simulink library "lib_all"  (Simulink 7.6)
settings: Solver=ode45  SolverMode=SingleTasking  StartTime=0.0  StopTime=10.0  RelTol=1e-3
values from neighbouring files (symbols this model uses):
  map_lib_03 = [6.8679 7.0159 7.4571 8.1849 9.1892 10.456 11.97 13.71 15.655 17.781 20.063 22.474 ... (803 elements, 73x11)]    [scr_simdata.mat]    (also in scr_simdata_all.mat)
  map_lib_06 = [2.5784 2.6727 2.9588 3.4453 4.1444 5.068 6.226 7.6242 9.2634 11.139 13.24 15.552 ... (803 elements, 73x11)]    [scr_simdata.mat]    (also in scr_simdata_all.mat)
  map_lib_09 = [0.0025784 0.0026727 0.0029588 0.0034453 0.0041444 0.005068 0.006226 0.0076242 0.0092634 0.011139 0.01324 0.015552 ... (803 elements, 73x11)]    [scr_simdata.mat]    (also in scr_simdata_all.mat)
  map_lib_10 = [2.74 2.75 2.76 2.77 2.78 2.79 2.8 2.81 2.82 2.83 2.84 2.85 ... (81 elements, 1x81)]    [scr_simdata.mat]    (also in scr_simdata_all.mat)
  map_lib_11 = [1.1983 1.2027 1.207 1.2113 1.2156 1.2198 1.2241 1.2283 1.2326 1.2368 1.241 1.2451 ... (81 elements, 1x81)]    [scr_simdata.mat]    (also in scr_simdata_all.mat)
  map_lib_12 = [2.74 2.75 2.76 2.77 2.78 2.79 2.8 2.81 2.82 2.83 2.84 2.85 ... (81 elements, 1x81)]    [scr_simdata.mat]    (also in scr_simdata_all.mat)
  map_lib_13 = [1.74 1.75 1.76 1.77 1.78 1.79 1.8 1.81 1.82 1.83 1.84 1.85 ... (81 elements, 1x81)]    [scr_simdata.mat]    (also in scr_simdata_all.mat)
  map_lib_16 = [0.00048828125 1.6159 1.8962 2.0821 2.2251 2.3426 2.4433 2.5318 2.611 2.683 2.749 2.8101 ... (1419 elements, 129x11)]    [scr_simdata.mat]    (also in scr_simdata_all.mat)
  map_mr2_01 = [0 800 1600 2400 3200 4000]    [scr_simdata.mat]    (also in scr_simdata_all.mat)
  map_mr2_02 = [1.32 1.32 1.33 1.34 1.36 1.38]    [scr_simdata.mat]    (also in scr_simdata_all.mat)
  map_mr2_03 = [0 800 1600 2400 3200 4000]    [scr_simdata.mat]    (also in scr_simdata_all.mat)
  map_mr2_04 = [1.32 1.32 1.33 1.34 1.36 1.38]    [scr_simdata.mat]    (also in scr_simdata_all.mat)
  map_mr2_05 = [0 800 1600 2400 3200 4000]    [scr_simdata.mat]    (also in scr_simdata_all.mat)
  map_mr2_06 = [1.32 1.32 1.33 1.34 1.36 1.38]    [scr_simdata.mat]    (also in scr_simdata_all.mat)
  Parameter1 = 6    [scr_simdata.mat]    (also in scr_simdata_all.mat)
  Parameter101 = 30    [scr_simdata.mat]    (also in scr_simdata_all.mat)
  Parameter102 = 12    [scr_simdata.mat]    (also in scr_simdata_all.mat)
  Parameter103 = -240    [scr_simdata.mat]    (also in scr_simdata_all.mat)
  Parameter108 = 48    [scr_simdata.mat]    (also in scr_simdata_all.mat)
  Parameter120 = 16    [scr_simdata.mat]    (also in scr_simdata_all.mat)
  Parameter121 = 87    [scr_simdata.mat]    (also in scr_simdata_all.mat)
  Parameter123 = 78    [scr_simdata.mat]    (also in scr_simdata_all.mat)
  Parameter124 = 60    [scr_simdata.mat]    (also in scr_simdata_all.mat)
  Parameter125 = -480    [scr_simdata.mat]    (also in scr_simdata_all.mat)
  Parameter130 = 96    [scr_simdata.mat]    (also in scr_simdata_all.mat)
  Parameter131 = 3    [scr_simdata.mat]    (also in scr_simdata_all.mat)
  Parameter144 = 3    [scr_simdata.mat]    (also in scr_simdata_all.mat)
  Parameter18 = 128    [scr_simdata.mat]    (also in scr_simdata_all.mat)
  Parameter19 = 16    [scr_simdata.mat]    (also in scr_simdata_all.mat)
  Parameter2 = 12    [scr_simdata.mat]    (also in scr_simdata_all.mat)
  Parameter20 = 7    [scr_simdata.mat]    (also in scr_simdata_all.mat)
  Parameter21 = 4    [scr_simdata.mat]    (also in scr_simdata_all.mat)
  Parameter3 = 18    [scr_simdata.mat]    (also in scr_simdata_all.mat)
  Parameter32 = 1    [scr_simdata.mat]    (also in scr_simdata_all.mat)
  Parameter34 = 24    [scr_simdata.mat]    (also in scr_simdata_all.mat)
  Parameter4 = 138    [scr_simdata.mat]    (also in scr_simdata_all.mat)
  Parameter42 = 48    [scr_simdata.mat]    (also in scr_simdata_all.mat)
  Parameter5 = 135    [scr_simdata.mat]    (also in scr_simdata_all.mat)
  Parameter55 = 0    [scr_simdata.mat]    (also in scr_simdata_all.mat)
  Parameter56 = 0    [scr_simdata.mat]    (also in scr_simdata_all.mat)
  Parameter57 = 1    [scr_simdata.mat]    (also in scr_simdata_all.mat)
  Parameter58 = 1    [scr_simdata.mat]    (also in scr_simdata_all.mat)
  Parameter59 = 2    [scr_simdata.mat]    (also in scr_simdata_all.mat)
  Parameter6 = 132    [scr_simdata.mat]    (also in scr_simdata_all.mat)
  Parameter60 = 2    [scr_simdata.mat]    (also in scr_simdata_all.mat)
  Parameter63 = 144    [scr_simdata.mat]    (also in scr_simdata_all.mat)
  Parameter7 = 126    [scr_simdata.mat]    (also in scr_simdata_all.mat)
  Parameter76 = 64    [scr_simdata.mat]    (also in scr_simdata_all.mat)
  Parameter77 = 135    [scr_simdata.mat]    (also in scr_simdata_all.mat)
  Parameter79 = 126    [scr_simdata.mat]    (also in scr_simdata_all.mat)
  Parameter80 = 108    [scr_simdata.mat]    (also in scr_simdata_all.mat)
  Parameter81 = 0    [scr_simdata.mat]    (also in scr_simdata_all.mat)
  Parameter86 = 0    [scr_simdata.mat]    (also in scr_simdata_all.mat)
  Parameter98 = 112    [scr_simdata.mat]    (also in scr_simdata_all.mat)
  Parameter99 = 39    [scr_simdata.mat]    (also in scr_simdata_all.mat)
undefined symbols (not defined in the file or in its folder): cylvolkp_hih_map2h, cylvolkp_map2h, pk1k_map2h
libraries: simulink x2
(part 18 of 36)

== system lib_all/SubSystem1364/SubSystem1419/SubSystem1428/SubSystem1452/SubSystem1453 ==
  note: ***編集禁止***
  element : Inport
  Inside_DSR : DataStoreRead  DataStoreName=DSM5
  Selector : Selector  IndexOptions=Index vector (port)  Indices=[1 3]  OutputSizes=1
  Output : Outport
  wires:
  Inside_DSR/1 -> Selector/1
  element/1 -> Selector/2
  Selector/1 -> Output/1

== system lib_all/SubSystem1364/SubSystem1419/SubSystem1428/SubSystem1452/SubSystem1454 ==
  Block3948 : Inport
  Block3949 : Inport  Port=2
  Block3950 : MultiPortSwitch  zeroidx=off  RndMeth=Zero  SaturateOnIntegerOverflow=on  InputSameDT=on
  SubSystem1455 : Subsystem  TreatAsAtomicUnit=on  mask(***ATC.DSRVEC***){bd_version=1.01.A, r0=DSM7, r1=-1, r2=, r3=, r4=, r5=, r6=, r7=, r8=, r9=, c0=, c1=, c2=, c3=, c4=, c5=, c6=, c7=, c8=, c9=}
      mask prompts: bd_version "Version"
      mask help: これはAutoCからのブロックです．編集禁止．
      settings: OpenFcn=warndlg('変更禁止!');
      mask Help: web(ui_blockhelpmng(gcb));
      mask Display: disp('ATC.DSRVEC')
  SubSystem1456 : Subsystem  TreatAsAtomicUnit=on  mask(***ATC.DSRVEC***){bd_version=1.01.A, r0=DSM6, r1=-1, r2=, r3=, r4=, r5=, r6=, r7=, r8=, r9=, c0=, c1=, c2=, c3=, c4=, c5=, c6=, c7=, c8=, c9=}
      mask prompts: bd_version "Version"
      mask help: これはAutoCからのブロックです．編集禁止．
      settings: OpenFcn=warndlg('変更禁止!');
      mask Help: web(ui_blockhelpmng(gcb));
      mask Display: disp('ATC.DSRVEC')
  SubSystem1457 : Subsystem  TreatAsAtomicUnit=on  mask(***ATC.DSRVEC***){bd_version=1.01.A, r0=DSM19, r1=-1, r2=, r3=, r4=, r5=, r6=, r7=, r8=, r9=, c0=, c1=, c2=, c3=, c4=, c5=, c6=, c7=, c8=, c9=}
      mask prompts: bd_version "Version"
      mask help: これはAutoCからのブロックです．編集禁止．
      settings: OpenFcn=warndlg('変更禁止!');
      mask Help: web(ui_blockhelpmng(gcb));
      mask Display: disp('ATC.DSRVEC')
  Block3951 : Outport
  wires:
  Block3949/1 -> SubSystem1457/1(element), SubSystem1456/1(element), SubSystem1455/1(element)
  SubSystem1457/1(Output) -> Block3950/4
  SubSystem1456/1(Output) -> Block3950/3
  SubSystem1455/1(Output) -> Block3950/2
  Block3950/1 -> Block3951/1
  Block3948/1 -> Block3950/1

== system lib_all/SubSystem1364/SubSystem1419/SubSystem1428/SubSystem1452/SubSystem1458 ==
  Block3952 : Inport
  Block3953 : Inport  Port=2
  Block3954 : MultiPortSwitch  zeroidx=off  RndMeth=Zero  SaturateOnIntegerOverflow=on  InputSameDT=on
  SubSystem1459 : Subsystem  TreatAsAtomicUnit=on  mask(***ATC.DSRVEC***){bd_version=1.01.A, r0=DSM7, r1=-1, r2=, r3=, r4=, r5=, r6=, r7=, r8=, r9=, c0=, c1=, c2=, c3=, c4=, c5=, c6=, c7=, c8=, c9=}
      mask prompts: bd_version "Version"
      mask help: これはAutoCからのブロックです．編集禁止．
      settings: OpenFcn=warndlg('変更禁止!');
      mask Help: web(ui_blockhelpmng(gcb));
      mask Display: disp('ATC.DSRVEC')
  SubSystem1460 : Subsystem  TreatAsAtomicUnit=on  mask(***ATC.DSRVEC***){bd_version=1.01.A, r0=DSM6, r1=-1, r2=, r3=, r4=, r5=, r6=, r7=, r8=, r9=, c0=, c1=, c2=, c3=, c4=, c5=, c6=, c7=, c8=, c9=}
      mask prompts: bd_version "Version"
      mask help: これはAutoCからのブロックです．編集禁止．
      settings: OpenFcn=warndlg('変更禁止!');
      mask Help: web(ui_blockhelpmng(gcb));
      mask Display: disp('ATC.DSRVEC')
  SubSystem1461 : Subsystem  TreatAsAtomicUnit=on  mask(***ATC.DSRVEC***){bd_version=1.01.A, r0=DSM19, r1=-1, r2=, r3=, r4=, r5=, r6=, r7=, r8=, r9=, c0=, c1=, c2=, c3=, c4=, c5=, c6=, c7=, c8=, c9=}
      mask prompts: bd_version "Version"
      mask help: これはAutoCからのブロックです．編集禁止．
      settings: OpenFcn=warndlg('変更禁止!');
      mask Help: web(ui_blockhelpmng(gcb));
      mask Display: disp('ATC.DSRVEC')
  Block3955 : Outport
  wires:
  Block3953/1 -> SubSystem1461/1(element), SubSystem1460/1(element), SubSystem1459/1(element)
  SubSystem1461/1(Output) -> Block3954/4
  SubSystem1460/1(Output) -> Block3954/3
  SubSystem1459/1(Output) -> Block3954/2
  Block3954/1 -> Block3955/1
  Block3952/1 -> Block3954/1

== system lib_all/SubSystem1364/SubSystem1419/SubSystem1428/SubSystem1462/SubSystem1463 ==
  note: ***編集禁止***
  element : Inport
  Inside_DSR : DataStoreRead  DataStoreName=DSM3
  Selector : Selector  IndexOptions=Index vector (port)  Indices=[1 3]  OutputSizes=1
  Output : Outport
  wires:
  Inside_DSR/1 -> Selector/1
  element/1 -> Selector/2
  Selector/1 -> Output/1

== system lib_all/SubSystem1364/SubSystem1419/SubSystem1428/SubSystem1462/SubSystem1464 ==
  Block3963 : Inport
  Block3964 : Inport  Port=2
  Block3965 : MultiPortSwitch  zeroidx=off  RndMeth=Zero  SaturateOnIntegerOverflow=on  InputSameDT=on
  SubSystem1465 : Subsystem  TreatAsAtomicUnit=on  mask(***ATC.DSRVEC***){bd_version=1.01.A, r0=DSM7, r1=-1, r2=, r3=, r4=, r5=, r6=, r7=, r8=, r9=, c0=, c1=, c2=, c3=, c4=, c5=, c6=, c7=, c8=, c9=}
      mask prompts: bd_version "Version"
      mask help: これはAutoCからのブロックです．編集禁止．
      settings: OpenFcn=warndlg('変更禁止!');
      mask Help: web(ui_blockhelpmng(gcb));
      mask Display: disp('ATC.DSRVEC')
  SubSystem1466 : Subsystem  TreatAsAtomicUnit=on  mask(***ATC.DSRVEC***){bd_version=1.01.A, r0=DSM6, r1=-1, r2=, r3=, r4=, r5=, r6=, r7=, r8=, r9=, c0=, c1=, c2=, c3=, c4=, c5=, c6=, c7=, c8=, c9=}
      mask prompts: bd_version "Version"
      mask help: これはAutoCからのブロックです．編集禁止．
      settings: OpenFcn=warndlg('変更禁止!');
      mask Help: web(ui_blockhelpmng(gcb));
      mask Display: disp('ATC.DSRVEC')
  SubSystem1467 : Subsystem  TreatAsAtomicUnit=on  mask(***ATC.DSRVEC***){bd_version=1.01.A, r0=DSM19, r1=-1, r2=, r3=, r4=, r5=, r6=, r7=, r8=, r9=, c0=, c1=, c2=, c3=, c4=, c5=, c6=, c7=, c8=, c9=}
      mask prompts: bd_version "Version"
      mask help: これはAutoCからのブロックです．編集禁止．
      settings: OpenFcn=warndlg('変更禁止!');
      mask Help: web(ui_blockhelpmng(gcb));
      mask Display: disp('ATC.DSRVEC')
  Block3966 : Outport
  wires:
  Block3964/1 -> SubSystem1467/1(element), SubSystem1466/1(element), SubSystem1465/1(element)
  SubSystem1467/1(Output) -> Block3965/4
  SubSystem1466/1(Output) -> Block3965/3
  SubSystem1465/1(Output) -> Block3965/2
  Block3965/1 -> Block3966/1
  Block3963/1 -> Block3965/1

== system lib_all/SubSystem1364/SubSystem1419/SubSystem1428/SubSystem1462/SubSystem1468 ==
  Block3967 : Inport
  Block3968 : Inport  Port=2
  Block3969 : MultiPortSwitch  zeroidx=off  RndMeth=Zero  SaturateOnIntegerOverflow=on  InputSameDT=on
  SubSystem1469 : Subsystem  TreatAsAtomicUnit=on  mask(***ATC.DSRVEC***){bd_version=1.01.A, r0=DSM7, r1=-1, r2=, r3=, r4=, r5=, r6=, r7=, r8=, r9=, c0=, c1=, c2=, c3=, c4=, c5=, c6=, c7=, c8=, c9=}
      mask prompts: bd_version "Version"
      mask help: これはAutoCからのブロックです．編集禁止．
      settings: OpenFcn=warndlg('変更禁止!');
      mask Help: web(ui_blockhelpmng(gcb));
      mask Display: disp('ATC.DSRVEC')
  SubSystem1470 : Subsystem  TreatAsAtomicUnit=on  mask(***ATC.DSRVEC***){bd_version=1.01.A, r0=DSM6, r1=-1, r2=, r3=, r4=, r5=, r6=, r7=, r8=, r9=, c0=, c1=, c2=, c3=, c4=, c5=, c6=, c7=, c8=, c9=}
      mask prompts: bd_version "Version"
      mask help: これはAutoCからのブロックです．編集禁止．
      settings: OpenFcn=warndlg('変更禁止!');
      mask Help: web(ui_blockhelpmng(gcb));
      mask Display: disp('ATC.DSRVEC')
  SubSystem1471 : Subsystem  TreatAsAtomicUnit=on  mask(***ATC.DSRVEC***){bd_version=1.01.A, r0=DSM19, r1=-1, r2=, r3=, r4=, r5=, r6=, r7=, r8=, r9=, c0=, c1=, c2=, c3=, c4=, c5=, c6=, c7=, c8=, c9=}
      mask prompts: bd_version "Version"
      mask help: これはAutoCからのブロックです．編集禁止．
      settings: OpenFcn=warndlg('変更禁止!');
      mask Help: web(ui_blockhelpmng(gcb));
      mask Display: disp('ATC.DSRVEC')
  Block3970 : Outport
  wires:
  Block3968/1 -> SubSystem1471/1(element), SubSystem1470/1(element), SubSystem1469/1(element)
  SubSystem1471/1(Output) -> Block3969/4
  SubSystem1470/1(Output) -> Block3969/3
  SubSystem1469/1(Output) -> Block3969/2
  Block3969/1 -> Block3970/1
  Block3967/1 -> Block3969/1

== system lib_all/SubSystem1487/SubSystem1496/SubSystem1501/SubSystem1502/SubSystem1503 ==
  note: ***編集禁止***
  element : Inport
  Inside_DSR : DataStoreRead  DataStoreName=DSM7
  Selector : Selector  IndexOptions=Index vector (port)  Indices=[1 3]  OutputSizes=1
  Output : Outport
  wires:
  Selector/1 -> Output/1
  element/1 -> Selector/2
  Inside_DSR/1 -> Selector/1

== system lib_all/SubSystem1487/SubSystem1496/SubSystem1501/SubSystem1502/SubSystem1504 ==
  note: ***編集禁止***
  element : Inport
  Inside_DSR : DataStoreRead  DataStoreName=DSM6
  Selector : Selector  IndexOptions=Index vector (port)  Indices=[1 3]  OutputSizes=1
  Output : Outport
  wires:
  Inside_DSR/1 -> Selector/1
  element/1 -> Selector/2
  Selector/1 -> Output/1

== system lib_all/SubSystem1487/SubSystem1496/SubSystem1501/SubSystem1502/SubSystem1505 ==
  note: ***編集禁止***
  element : Inport
  Inside_DSR : DataStoreRead  DataStoreName=DSM19
  Selector : Selector  IndexOptions=Index vector (port)  Indices=[1 3]  OutputSizes=1
  Output : Outport
  wires:
  Selector/1 -> Output/1
  element/1 -> Selector/2
  Inside_DSR/1 -> Selector/1

== system lib_all/SubSystem1487/SubSystem1496/SubSystem1501/SubSystem1506/SubSystem1507 ==
  note: ***編集禁止***
  element : Inport
  Inside_DSR : DataStoreRead  DataStoreName=DSM7
  Selector : Selector  IndexOptions=Index vector (port)  Indices=[1 3]  OutputSizes=1
  Output : Outport
  wires:
  Selector/1 -> Output/1
  element/1 -> Selector/2
  Inside_DSR/1 -> Selector/1

== system lib_all/SubSystem1487/SubSystem1496/SubSystem1501/SubSystem1506/SubSystem1508 ==
  note: ***編集禁止***
  element : Inport
  Inside_DSR : DataStoreRead  DataStoreName=DSM6
  Selector : Selector  IndexOptions=Index vector (port)  Indices=[1 3]  OutputSizes=1
  Output : Outport
  wires:
  Inside_DSR/1 -> Selector/1
  element/1 -> Selector/2
  Selector/1 -> Output/1

== system lib_all/SubSystem1487/SubSystem1496/SubSystem1501/SubSystem1506/SubSystem1509 ==
  note: ***編集禁止***
  element : Inport
  Inside_DSR : DataStoreRead  DataStoreName=DSM19
  Selector : Selector  IndexOptions=Index vector (port)  Indices=[1 3]  OutputSizes=1
  Output : Outport
  wires:
  Selector/1 -> Output/1
  element/1 -> Selector/2
  Inside_DSR/1 -> Selector/1

== system lib_all/SubSystem1487/SubSystem1496/SubSystem1510/SubSystem1512/SubSystem1513 ==
  note: ***編集禁止***
  element : Inport
  Inside_DSR : DataStoreRead  DataStoreName=DSM7
  Selector : Selector  IndexOptions=Index vector (port)  Indices=[1 3]  OutputSizes=1
  Output : Outport
  wires:
  Selector/1 -> Output/1
  element/1 -> Selector/2
  Inside_DSR/1 -> Selector/1

== system lib_all/SubSystem1487/SubSystem1496/SubSystem1510/SubSystem1512/SubSystem1514 ==
  note: ***編集禁止***
  element : Inport
  Inside_DSR : DataStoreRead  DataStoreName=DSM6
  Selector : Selector  IndexOptions=Index vector (port)  Indices=[1 3]  OutputSizes=1
  Output : Outport
  wires:
  Inside_DSR/1 -> Selector/1
  element/1 -> Selector/2
  Selector/1 -> Output/1

== system lib_all/SubSystem1487/SubSystem1496/SubSystem1510/SubSystem1512/SubSystem1515 ==
  note: ***編集禁止***
  element : Inport
  Inside_DSR : DataStoreRead  DataStoreName=DSM19
  Selector : Selector  IndexOptions=Index vector (port)  Indices=[1 3]  OutputSizes=1
  Output : Outport
  wires:
  Selector/1 -> Output/1
  element/1 -> Selector/2
  Inside_DSR/1 -> Selector/1

== system lib_all/SubSystem1487/SubSystem1496/SubSystem1510/SubSystem1516/SubSystem1517 ==
  note: ***編集禁止***
  element : Inport
  Inside_DSR : DataStoreRead  DataStoreName=DSM7
  Selector : Selector  IndexOptions=Index vector (port)  Indices=[1 3]  OutputSizes=1
  Output : Outport
  wires:
  Selector/1 -> Output/1
  element/1 -> Selector/2
  Inside_DSR/1 -> Selector/1

== system lib_all/SubSystem1487/SubSystem1496/SubSystem1510/SubSystem1516/SubSystem1518 ==
  note: ***編集禁止***
  element : Inport
  Inside_DSR : DataStoreRead  DataStoreName=DSM6
  Selector : Selector  IndexOptions=Index vector (port)  Indices=[1 3]  OutputSizes=1
  Output : Outport
  wires:
  Inside_DSR/1 -> Selector/1
  element/1 -> Selector/2
  Selector/1 -> Output/1

== system lib_all/SubSystem1487/SubSystem1496/SubSystem1510/SubSystem1516/SubSystem1519 ==
  note: ***編集禁止***
  element : Inport
  Inside_DSR : DataStoreRead  DataStoreName=DSM19
  Selector : Selector  IndexOptions=Index vector (port)  Indices=[1 3]  OutputSizes=1
  Output : Outport
  wires:
  Selector/1 -> Output/1
  element/1 -> Selector/2
  Inside_DSR/1 -> Selector/1

== system lib_all/SubSystem1487/SubSystem1496/SubSystem1520/SubSystem1522/SubSystem1523 ==
  note: ***編集禁止***
  element : Inport
  Inside_DSR : DataStoreRead  DataStoreName=DSM7
  Selector : Selector  IndexOptions=Index vector (port)  Indices=[1 3]  OutputSizes=1
  Output : Outport
  wires:
  Selector/1 -> Output/1
  element/1 -> Selector/2
  Inside_DSR/1 -> Selector/1

== system lib_all/SubSystem1487/SubSystem1496/SubSystem1520/SubSystem1522/SubSystem1524 ==
  note: ***編集禁止***
  element : Inport
  Inside_DSR : DataStoreRead  DataStoreName=DSM6
  Selector : Selector  IndexOptions=Index vector (port)  Indices=[1 3]  OutputSizes=1
  Output : Outport
  wires:
  Inside_DSR/1 -> Selector/1
  element/1 -> Selector/2
  Selector/1 -> Output/1

== system lib_all/SubSystem1487/SubSystem1496/SubSystem1520/SubSystem1522/SubSystem1525 ==
  note: ***編集禁止***
  element : Inport
  Inside_DSR : DataStoreRead  DataStoreName=DSM19
  Selector : Selector  IndexOptions=Index vector (port)  Indices=[1 3]  OutputSizes=1
  Output : Outport
  wires:
  Selector/1 -> Output/1
  element/1 -> Selector/2
  Inside_DSR/1 -> Selector/1

== system lib_all/SubSystem1487/SubSystem1496/SubSystem1520/SubSystem1526/SubSystem1527 ==
  note: ***編集禁止***
  element : Inport
  Inside_DSR : DataStoreRead  DataStoreName=DSM7
  Selector : Selector  IndexOptions=Index vector (port)  Indices=[1 3]  OutputSizes=1
  Output : Outport
  wires:
  Selector/1 -> Output/1
  element/1 -> Selector/2
  Inside_DSR/1 -> Selector/1

== system lib_all/SubSystem1487/SubSystem1496/SubSystem1520/SubSystem1526/SubSystem1528 ==
  note: ***編集禁止***
  element : Inport
  Inside_DSR : DataStoreRead  DataStoreName=DSM6
  Selector : Selector  IndexOptions=Index vector (port)  Indices=[1 3]  OutputSizes=1
  Output : Outport
  wires:
  Inside_DSR/1 -> Selector/1
  element/1 -> Selector/2
  Selector/1 -> Output/1

== system lib_all/SubSystem1487/SubSystem1496/SubSystem1520/SubSystem1526/SubSystem1529 ==
  note: ***編集禁止***
  element : Inport
  Inside_DSR : DataStoreRead  DataStoreName=DSM19
  Selector : Selector  IndexOptions=Index vector (port)  Indices=[1 3]  OutputSizes=1
  Output : Outport
  wires:
  Selector/1 -> Output/1
  element/1 -> Selector/2
  Inside_DSR/1 -> Selector/1

== system lib_all/SubSystem1487/SubSystem1496/SubSystem1530/SubSystem1532/SubSystem1533 ==
  note: ***編集禁止***
  element : Inport
  Inside_DSR : DataStoreRead  DataStoreName=DSM7
  Selector : Selector  IndexOptions=Index vector (port)  Indices=[1 3]  OutputSizes=1
  Output : Outport
  wires:
  Selector/1 -> Output/1
  element/1 -> Selector/2
  Inside_DSR/1 -> Selector/1

== system lib_all/SubSystem1487/SubSystem1496/SubSystem1530/SubSystem1532/SubSystem1534 ==
  note: ***編集禁止***
  element : Inport
  Inside_DSR : DataStoreRead  DataStoreName=DSM6
  Selector : Selector  IndexOptions=Index vector (port)  Indices=[1 3]  OutputSizes=1
  Output : Outport
  wires:
  Inside_DSR/1 -> Selector/1
  element/1 -> Selector/2
  Selector/1 -> Output/1

== system lib_all/SubSystem1487/SubSystem1496/SubSystem1530/SubSystem1532/SubSystem1535 ==
  note: ***編集禁止***
  element : Inport
  Inside_DSR : DataStoreRead  DataStoreName=DSM19
  Selector : Selector  IndexOptions=Index vector (port)  Indices=[1 3]  OutputSizes=1
  Output : Outport
  wires:
  Selector/1 -> Output/1
  element/1 -> Selector/2
  Inside_DSR/1 -> Selector/1

== system lib_all/SubSystem1487/SubSystem1496/SubSystem1530/SubSystem1536/SubSystem1537 ==
  note: ***編集禁止***
  element : Inport
  Inside_DSR : DataStoreRead  DataStoreName=DSM7
  Selector : Selector  IndexOptions=Index vector (port)  Indices=[1 3]  OutputSizes=1
  Output : Outport
  wires:
  Selector/1 -> Output/1
  element/1 -> Selector/2
  Inside_DSR/1 -> Selector/1

== system lib_all/SubSystem1487/SubSystem1496/SubSystem1530/SubSystem1536/SubSystem1538 ==
  note: ***編集禁止***
  element : Inport
  Inside_DSR : DataStoreRead  DataStoreName=DSM6
  Selector : Selector  IndexOptions=Index vector (port)  Indices=[1 3]  OutputSizes=1
  Output : Outport
  wires:
  Inside_DSR/1 -> Selector/1
  element/1 -> Selector/2
  Selector/1 -> Output/1

== system lib_all/SubSystem1487/SubSystem1496/SubSystem1530/SubSystem1536/SubSystem1539 ==
  note: ***編集禁止***
  element : Inport
  Inside_DSR : DataStoreRead  DataStoreName=DSM19
  Selector : Selector  IndexOptions=Index vector (port)  Indices=[1 3]  OutputSizes=1
  Output : Outport
  wires:
  Selector/1 -> Output/1
  element/1 -> Selector/2
  Inside_DSR/1 -> Selector/1

== system lib_all/SubSystem1540/SubSystem1549/SubSystem1554/SubSystem1555/SubSystem1556 ==
  note: ***編集禁止***
  element : Inport
  Inside_DSR : DataStoreRead  DataStoreName=DSM7
  Selector : Selector  IndexOptions=Index vector (port)  Indices=[1 3]  OutputSizes=1
  Output : Outport
  wires:
  Selector/1 -> Output/1
  element/1 -> Selector/2
  Inside_DSR/1 -> Selector/1

== system lib_all/SubSystem1540/SubSystem1549/SubSystem1554/SubSystem1555/SubSystem1557 ==
  note: ***編集禁止***
  element : Inport
  Inside_DSR : DataStoreRead  DataStoreName=DSM6
  Selector : Selector  IndexOptions=Index vector (port)  Indices=[1 3]  OutputSizes=1
  Output : Outport
  wires:
  Inside_DSR/1 -> Selector/1
  element/1 -> Selector/2
  Selector/1 -> Output/1

== system lib_all/SubSystem1540/SubSystem1549/SubSystem1554/SubSystem1555/SubSystem1558 ==
  note: ***編集禁止***
  element : Inport
  Inside_DSR : DataStoreRead  DataStoreName=DSM19
  Selector : Selector  IndexOptions=Index vector (port)  Indices=[1 3]  OutputSizes=1
  Output : Outport
  wires:
  Selector/1 -> Output/1
  element/1 -> Selector/2
  Inside_DSR/1 -> Selector/1

== system lib_all/SubSystem1540/SubSystem1549/SubSystem1554/SubSystem1559/SubSystem1560 ==
  note: ***編集禁止***
  element : Inport
  Inside_DSR : DataStoreRead  DataStoreName=DSM7
  Selector : Selector  IndexOptions=Index vector (port)  Indices=[1 3]  OutputSizes=1
  Output : Outport
  wires:
  Selector/1 -> Output/1
  element/1 -> Selector/2
  Inside_DSR/1 -> Selector/1

== system lib_all/SubSystem1540/SubSystem1549/SubSystem1554/SubSystem1559/SubSystem1561 ==
  note: ***編集禁止***
  element : Inport
  Inside_DSR : DataStoreRead  DataStoreName=DSM6
  Selector : Selector  IndexOptions=Index vector (port)  Indices=[1 3]  OutputSizes=1
  Output : Outport
  wires:
  Inside_DSR/1 -> Selector/1
  element/1 -> Selector/2
  Selector/1 -> Output/1

== system lib_all/SubSystem1540/SubSystem1549/SubSystem1554/SubSystem1559/SubSystem1562 ==
  note: ***編集禁止***
  element : Inport
  Inside_DSR : DataStoreRead  DataStoreName=DSM19
  Selector : Selector  IndexOptions=Index vector (port)  Indices=[1 3]  OutputSizes=1
  Output : Outport
  wires:
  Selector/1 -> Output/1
  element/1 -> Selector/2
  Inside_DSR/1 -> Selector/1

== system lib_all/SubSystem1540/SubSystem1549/SubSystem1563/SubSystem1565/SubSystem1566 ==
  note: ***編集禁止***
  element : Inport
  Inside_DSR : DataStoreRead  DataStoreName=DSM7
  Selector : Selector  IndexOptions=Index vector (port)  Indices=[1 3]  OutputSizes=1
  Output : Outport
  wires:
  Selector/1 -> Output/1
  element/1 -> Selector/2
  Inside_DSR/1 -> Selector/1

== system lib_all/SubSystem1540/SubSystem1549/SubSystem1563/SubSystem1565/SubSystem1567 ==
  note: ***編集禁止***
  element : Inport
  Inside_DSR : DataStoreRead  DataStoreName=DSM6
  Selector : Selector  IndexOptions=Index vector (port)  Indices=[1 3]  OutputSizes=1
  Output : Outport
  wires:
  Inside_DSR/1 -> Selector/1
  element/1 -> Selector/2
  Selector/1 -> Output/1

== system lib_all/SubSystem1540/SubSystem1549/SubSystem1563/SubSystem1565/SubSystem1568 ==
  note: ***編集禁止***
  element : Inport
  Inside_DSR : DataStoreRead  DataStoreName=DSM19
  Selector : Selector  IndexOptions=Index vector (port)  Indices=[1 3]  OutputSizes=1
  Output : Outport
  wires:
  Selector/1 -> Output/1
  element/1 -> Selector/2
  Inside_DSR/1 -> Selector/1

== system lib_all/SubSystem1540/SubSystem1549/SubSystem1563/SubSystem1569/SubSystem1570 ==
  note: ***編集禁止***
  element : Inport
  Inside_DSR : DataStoreRead  DataStoreName=DSM7
  Selector : Selector  IndexOptions=Index vector (port)  Indices=[1 3]  OutputSizes=1
  Output : Outport
  wires:
  Selector/1 -> Output/1
  element/1 -> Selector/2
  Inside_DSR/1 -> Selector/1

== system lib_all/SubSystem1540/SubSystem1549/SubSystem1563/SubSystem1569/SubSystem1571 ==
  note: ***編集禁止***
  element : Inport
  Inside_DSR : DataStoreRead  DataStoreName=DSM6
  Selector : Selector  IndexOptions=Index vector (port)  Indices=[1 3]  OutputSizes=1
  Output : Outport
  wires:
  Inside_DSR/1 -> Selector/1
  element/1 -> Selector/2
  Selector/1 -> Output/1

== system lib_all/SubSystem1540/SubSystem1549/SubSystem1563/SubSystem1569/SubSystem1572 ==
  note: ***編集禁止***
  element : Inport
  Inside_DSR : DataStoreRead  DataStoreName=DSM19
  Selector : Selector  IndexOptions=Index vector (port)  Indices=[1 3]  OutputSizes=1
  Output : Outport
  wires:
  Selector/1 -> Output/1
  element/1 -> Selector/2
  Inside_DSR/1 -> Selector/1

== system lib_all/SubSystem1540/SubSystem1549/SubSystem1573/SubSystem1575/SubSystem1576 ==
  note: ***編集禁止***
  element : Inport
  Inside_DSR : DataStoreRead  DataStoreName=DSM7
  Selector : Selector  IndexOptions=Index vector (port)  Indices=[1 3]  OutputSizes=1
  Output : Outport
  wires:
  Selector/1 -> Output/1
  element/1 -> Selector/2
  Inside_DSR/1 -> Selector/1

== system lib_all/SubSystem1540/SubSystem1549/SubSystem1573/SubSystem1575/SubSystem1577 ==
  note: ***編集禁止***
  element : Inport
  Inside_DSR : DataStoreRead  DataStoreName=DSM6
  Selector : Selector  IndexOptions=Index vector (port)  Indices=[1 3]  OutputSizes=1
  Output : Outport
  wires:
  Inside_DSR/1 -> Selector/1
  element/1 -> Selector/2
  Selector/1 -> Output/1

== system lib_all/SubSystem1540/SubSystem1549/SubSystem1573/SubSystem1575/SubSystem1578 ==
  note: ***編集禁止***
  element : Inport
  Inside_DSR : DataStoreRead  DataStoreName=DSM19
  Selector : Selector  IndexOptions=Index vector (port)  Indices=[1 3]  OutputSizes=1
  Output : Outport
  wires:
  Selector/1 -> Output/1
  element/1 -> Selector/2
  Inside_DSR/1 -> Selector/1

== system lib_all/SubSystem1540/SubSystem1549/SubSystem1573/SubSystem1579/SubSystem1580 ==
  note: ***編集禁止***
  element : Inport
  Inside_DSR : DataStoreRead  DataStoreName=DSM7
  Selector : Selector  IndexOptions=Index vector (port)  Indices=[1 3]  OutputSizes=1
  Output : Outport
  wires:
  Selector/1 -> Output/1
  element/1 -> Selector/2
  Inside_DSR/1 -> Selector/1

== system lib_all/SubSystem1540/SubSystem1549/SubSystem1573/SubSystem1579/SubSystem1581 ==
  note: ***編集禁止***
  element : Inport
  Inside_DSR : DataStoreRead  DataStoreName=DSM6
  Selector : Selector  IndexOptions=Index vector (port)  Indices=[1 3]  OutputSizes=1
  Output : Outport
  wires:
  Inside_DSR/1 -> Selector/1
  element/1 -> Selector/2
  Selector/1 -> Output/1

== system lib_all/SubSystem1540/SubSystem1549/SubSystem1573/SubSystem1579/SubSystem1582 ==
  note: ***編集禁止***
  element : Inport
  Inside_DSR : DataStoreRead  DataStoreName=DSM19
  Selector : Selector  IndexOptions=Index vector (port)  Indices=[1 3]  OutputSizes=1
  Output : Outport
  wires:
  Selector/1 -> Output/1
  element/1 -> Selector/2
  Inside_DSR/1 -> Selector/1

== system lib_all/SubSystem1540/SubSystem1549/SubSystem1583/SubSystem1585/SubSystem1586 ==
  note: ***編集禁止***
  element : Inport
  Inside_DSR : DataStoreRead  DataStoreName=DSM7
  Selector : Selector  IndexOptions=Index vector (port)  Indices=[1 3]  OutputSizes=1
  Output : Outport
  wires:
  Selector/1 -> Output/1
  element/1 -> Selector/2
  Inside_DSR/1 -> Selector/1

== system lib_all/SubSystem1540/SubSystem1549/SubSystem1583/SubSystem1585/SubSystem1587 ==
  note: ***編集禁止***
  element : Inport
  Inside_DSR : DataStoreRead  DataStoreName=DSM6
  Selector : Selector  IndexOptions=Index vector (port)  Indices=[1 3]  OutputSizes=1
  Output : Outport
  wires:
  Inside_DSR/1 -> Selector/1
  element/1 -> Selector/2
  Selector/1 -> Output/1

== system lib_all/SubSystem1540/SubSystem1549/SubSystem1583/SubSystem1585/SubSystem1588 ==
  note: ***編集禁止***
  element : Inport
  Inside_DSR : DataStoreRead  DataStoreName=DSM19
  Selector : Selector  IndexOptions=Index vector (port)  Indices=[1 3]  OutputSizes=1
  Output : Outport
  wires:
  Selector/1 -> Output/1
  element/1 -> Selector/2
  Inside_DSR/1 -> Selector/1

== system lib_all/SubSystem1540/SubSystem1549/SubSystem1583/SubSystem1589/SubSystem1590 ==
  note: ***編集禁止***
  element : Inport
  Inside_DSR : DataStoreRead  DataStoreName=DSM7
  Selector : Selector  IndexOptions=Index vector (port)  Indices=[1 3]  OutputSizes=1
  Output : Outport
  wires:
  Selector/1 -> Output/1
  element/1 -> Selector/2
  Inside_DSR/1 -> Selector/1

== system lib_all/SubSystem1540/SubSystem1549/SubSystem1583/SubSystem1589/SubSystem1591 ==
  note: ***編集禁止***
  element : Inport
  Inside_DSR : DataStoreRead  DataStoreName=DSM6
  Selector : Selector  IndexOptions=Index vector (port)  Indices=[1 3]  OutputSizes=1
  Output : Outport
  wires:
  Inside_DSR/1 -> Selector/1
  element/1 -> Selector/2
  Selector/1 -> Output/1

== system lib_all/SubSystem1540/SubSystem1549/SubSystem1583/SubSystem1589/SubSystem1592 ==
  note: ***編集禁止***
  element : Inport
  Inside_DSR : DataStoreRead  DataStoreName=DSM19
  Selector : Selector  IndexOptions=Index vector (port)  Indices=[1 3]  OutputSizes=1
  Output : Outport
  wires:
  Selector/1 -> Output/1
  element/1 -> Selector/2
  Inside_DSR/1 -> Selector/1

== system lib_all/SubSystem1593/SubSystem1595/SubSystem1598/SubSystem1599/Product ==
  note: ***編集禁止***
  mult : Inport
  div : Inport  Port=2
  init : Inport  Port=3
  Constant1 : Constant  OutDataTypeStr=Inherit: Inherit via back propagation
  Constant2 : Constant  Value=0  OutDataTypeStr=Inherit: Inherit via back propagation
  Product : Product  Inputs=*/  SaturateOnIntegerOverflow=on  RndMeth=Zero  InputSameDT=on  OutDataTypeStr=Inherit: Same as first input
  Relational Operator : RelationalOperator  Operator=~=  InputSameDT=on
  Switch : Switch  Threshold=0.5  SaturateOnIntegerOverflow=on  InputSameDT=on
  Switch1 : Switch  Threshold=0.5  SaturateOnIntegerOverflow=on  InputSameDT=on
  Out : Outport
  wires:
  mult/1 -> Product/1
  Product/1 -> Switch/1
  Relational Operator/1 -> Switch/2, Switch1/2
  init/1 -> Switch/3
  Switch/1 -> Out/1
  div/1 -> Switch1/1, Relational Operator/1
  Switch1/1 -> Product/2
  Constant2/1 -> Relational Operator/2
  Constant1/1 -> Switch1/3

== system lib_all/SubSystem1593/SubSystem1595/SubSystem1598/SubSystem1601/SubSystem1602 ==
  note: ***編集禁止***
  In1 : Inport
  In2 : Inport  Port=2
  RO1 : RelationalOperator  Operator=>  InputSameDT=on
  single1 : DataTypeConversion  OutDataTypeStr=single  SaturateOnIntegerOverflow=on  RndMeth=Zero
  single2 : DataTypeConversion  OutDataTypeStr=single  SaturateOnIntegerOverflow=on  RndMeth=Zero
  Out1 : Outport
  wires:
  RO1/1 -> Out1/1
  In2/1 -> single2/1
  In1/1 -> single1/1
  single1/1 -> RO1/1
  single2/1 -> RO1/2

== system lib_all/SubSystem1593/SubSystem1595/SubSystem1598/SubSystem1601/SubSystem1603 ==
  note: ***編集禁止***
  In1 : Inport
  In2 : Inport  Port=2
  LO1 : Logic
  RO1 : RelationalOperator  Operator=<  InputSameDT=on
  RO2 : RelationalOperator  Operator=~=  InputSameDT=on
  single1 : DataTypeConversion  OutDataTypeStr=single  SaturateOnIntegerOverflow=on  RndMeth=Zero
  single2 : DataTypeConversion  OutDataTypeStr=single  SaturateOnIntegerOverflow=on  RndMeth=Zero
  Out1 : Outport
  wires:
  In2/1 -> single2/1
  In1/1 -> single1/1
  single1/1 -> RO1/1, RO2/1
  single2/1 -> RO1/2, RO2/2
  RO1/1 -> LO1/1
  RO2/1 -> LO1/2
  LO1/1 -> Out1/1

== system lib_all/SubSystem1593/SubSystem1595/SubSystem1604/SubSystem1609/SubSystem1610 ==
  Block4335 : Inport
  Block4336 : Inport  Port=2
  Block4337 : MultiPortSwitch  zeroidx=off  RndMeth=Zero  SaturateOnIntegerOverflow=on  InputSameDT=on
  SubSystem1611 : Subsystem  TreatAsAtomicUnit=on  mask(***ATC.DSRVEC***){bd_version=1.01.A, r0=DSM7, r1=-1, r2=, r3=, r4=, r5=, r6=, r7=, r8=, r9=, c0=, c1=, c2=, c3=, c4=, c5=, c6=, c7=, c8=, c9=}
      mask prompts: bd_version "Version"
      mask help: これはAutoCからのブロックです．編集禁止．
      settings: OpenFcn=warndlg('変更禁止!');
      mask Help: web(ui_blockhelpmng(gcb));
      mask Display: disp('ATC.DSRVEC')
  SubSystem1612 : Subsystem  TreatAsAtomicUnit=on  mask(***ATC.DSRVEC***){bd_version=1.01.A, r0=DSM6, r1=-1, r2=, r3=, r4=, r5=, r6=, r7=, r8=, r9=, c0=, c1=, c2=, c3=, c4=, c5=, c6=, c7=, c8=, c9=}
      mask prompts: bd_version "Version"
      mask help: これはAutoCからのブロックです．編集禁止．
      settings: OpenFcn=warndlg('変更禁止!');
      mask Help: web(ui_blockhelpmng(gcb));
      mask Display: disp('ATC.DSRVEC')
  SubSystem1613 : Subsystem  TreatAsAtomicUnit=on  mask(***ATC.DSRVEC***){bd_version=1.01.A, r0=DSM19, r1=-1, r2=, r3=, r4=, r5=, r6=, r7=, r8=, r9=, c0=, c1=, c2=, c3=, c4=, c5=, c6=, c7=, c8=, c9=}
      mask prompts: bd_version "Version"
      mask help: これはAutoCからのブロックです．編集禁止．
      settings: OpenFcn=warndlg('変更禁止!');
      mask Help: web(ui_blockhelpmng(gcb));
      mask Display: disp('ATC.DSRVEC')
  Block4338 : Outport
  wires:
  Block4335/1 -> Block4337/1
  Block4337/1 -> Block4338/1
  SubSystem1611/1(Output) -> Block4337/2
  SubSystem1612/1(Output) -> Block4337/3
  SubSystem1613/1(Output) -> Block4337/4
  Block4336/1 -> SubSystem1611/1(element), SubSystem1612/1(element), SubSystem1613/1(element)

== system lib_all/SubSystem1593/SubSystem1595/SubSystem1604/SubSystem1609/SubSystem1614 ==
  Block4339 : Inport
  Block4340 : Inport  Port=2
  Block4341 : MultiPortSwitch  zeroidx=off  RndMeth=Zero  SaturateOnIntegerOverflow=on  InputSameDT=on
  SubSystem1615 : Subsystem  TreatAsAtomicUnit=on  mask(***ATC.DSRVEC***){bd_version=1.01.A, r0=DSM7, r1=-1, r2=, r3=, r4=, r5=, r6=, r7=, r8=, r9=, c0=, c1=, c2=, c3=, c4=, c5=, c6=, c7=, c8=, c9=}
      mask prompts: bd_version "Version"
      mask help: これはAutoCからのブロックです．編集禁止．
      settings: OpenFcn=warndlg('変更禁止!');
      mask Help: web(ui_blockhelpmng(gcb));
      mask Display: disp('ATC.DSRVEC')
  SubSystem1616 : Subsystem  TreatAsAtomicUnit=on  mask(***ATC.DSRVEC***){bd_version=1.01.A, r0=DSM6, r1=-1, r2=, r3=, r4=, r5=, r6=, r7=, r8=, r9=, c0=, c1=, c2=, c3=, c4=, c5=, c6=, c7=, c8=, c9=}
      mask prompts: bd_version "Version"
      mask help: これはAutoCからのブロックです．編集禁止．
      settings: OpenFcn=warndlg('変更禁止!');
      mask Help: web(ui_blockhelpmng(gcb));
      mask Display: disp('ATC.DSRVEC')
  SubSystem1617 : Subsystem  TreatAsAtomicUnit=on  mask(***ATC.DSRVEC***){bd_version=1.01.A, r0=DSM19, r1=-1, r2=, r3=, r4=, r5=, r6=, r7=, r8=, r9=, c0=, c1=, c2=, c3=, c4=, c5=, c6=, c7=, c8=, c9=}
      mask prompts: bd_version "Version"
      mask help: これはAutoCからのブロックです．編集禁止．
      settings: OpenFcn=warndlg('変更禁止!');
      mask Help: web(ui_blockhelpmng(gcb));
      mask Display: disp('ATC.DSRVEC')
  Block4342 : Outport
  wires:
  Block4339/1 -> Block4341/1
  Block4341/1 -> Block4342/1
  SubSystem1615/1(Output) -> Block4341/2
  SubSystem1616/1(Output) -> Block4341/3
  SubSystem1617/1(Output) -> Block4341/4
  Block4340/1 -> SubSystem1615/1(element), SubSystem1616/1(element), SubSystem1617/1(element)

== system lib_all/SubSystem1593/SubSystem1595/SubSystem1604/SubSystem1618/SubSystem1619 ==
  note: ***編集禁止***
  element : Inport
  Inside_DSR : DataStoreRead  DataStoreName=DSM1
  Selector : Selector  IndexOptions=Index vector (port)  Indices=[1 3]  OutputSizes=1
  Output : Outport
  wires:
  Selector/1 -> Output/1
  element/1 -> Selector/2
  Inside_DSR/1 -> Selector/1

== system lib_all/SubSystem1593/SubSystem1595/SubSystem1604/SubSystem1618/SubSystem1620 ==
  Block4350 : Inport
  Block4351 : Inport  Port=2
  Block4352 : MultiPortSwitch  zeroidx=off  RndMeth=Zero  SaturateOnIntegerOverflow=on  InputSameDT=on
  SubSystem1621 : Subsystem  TreatAsAtomicUnit=on  mask(***ATC.DSRVEC***){bd_version=1.01.A, r0=DSM7, r1=-1, r2=, r3=, r4=, r5=, r6=, r7=, r8=, r9=, c0=, c1=, c2=, c3=, c4=, c5=, c6=, c7=, c8=, c9=}
      mask prompts: bd_version "Version"
      mask help: これはAutoCからのブロックです．編集禁止．
      settings: OpenFcn=warndlg('変更禁止!');
      mask Help: web(ui_blockhelpmng(gcb));
      mask Display: disp('ATC.DSRVEC')
  SubSystem1622 : Subsystem  TreatAsAtomicUnit=on  mask(***ATC.DSRVEC***){bd_version=1.01.A, r0=DSM6, r1=-1, r2=, r3=, r4=, r5=, r6=, r7=, r8=, r9=, c0=, c1=, c2=, c3=, c4=, c5=, c6=, c7=, c8=, c9=}
      mask prompts: bd_version "Version"
      mask help: これはAutoCからのブロックです．編集禁止．
      settings: OpenFcn=warndlg('変更禁止!');
      mask Help: web(ui_blockhelpmng(gcb));
      mask Display: disp('ATC.DSRVEC')
  SubSystem1623 : Subsystem  TreatAsAtomicUnit=on  mask(***ATC.DSRVEC***){bd_version=1.01.A, r0=DSM19, r1=-1, r2=, r3=, r4=, r5=, r6=, r7=, r8=, r9=, c0=, c1=, c2=, c3=, c4=, c5=, c6=, c7=, c8=, c9=}
      mask prompts: bd_version "Version"
      mask help: これはAutoCからのブロックです．編集禁止．
      settings: OpenFcn=warndlg('変更禁止!');
      mask Help: web(ui_blockhelpmng(gcb));
      mask Display: disp('ATC.DSRVEC')
  Block4353 : Outport
  wires:
  Block4350/1 -> Block4352/1
  Block4352/1 -> Block4353/1
  SubSystem1621/1(Output) -> Block4352/2
  SubSystem1622/1(Output) -> Block4352/3
  SubSystem1623/1(Output) -> Block4352/4
  Block4351/1 -> SubSystem1621/1(element), SubSystem1622/1(element), SubSystem1623/1(element)

== system lib_all/SubSystem1593/SubSystem1595/SubSystem1604/SubSystem1618/SubSystem1624 ==
  Block4354 : Inport
  Block4355 : Inport  Port=2
  Block4356 : MultiPortSwitch  zeroidx=off  RndMeth=Zero  SaturateOnIntegerOverflow=on  InputSameDT=on
  SubSystem1625 : Subsystem  TreatAsAtomicUnit=on  mask(***ATC.DSRVEC***){bd_version=1.01.A, r0=DSM7, r1=-1, r2=, r3=, r4=, r5=, r6=, r7=, r8=, r9=, c0=, c1=, c2=, c3=, c4=, c5=, c6=, c7=, c8=, c9=}
      mask prompts: bd_version "Version"
      mask help: これはAutoCからのブロックです．編集禁止．
      settings: OpenFcn=warndlg('変更禁止!');
      mask Help: web(ui_blockhelpmng(gcb));
      mask Display: disp('ATC.DSRVEC')
  SubSystem1626 : Subsystem  TreatAsAtomicUnit=on  mask(***ATC.DSRVEC***){bd_version=1.01.A, r0=DSM6, r1=-1, r2=, r3=, r4=, r5=, r6=, r7=, r8=, r9=, c0=, c1=, c2=, c3=, c4=, c5=, c6=, c7=, c8=, c9=}
      mask prompts: bd_version "Version"
      mask help: これはAutoCからのブロックです．編集禁止．
      settings: OpenFcn=warndlg('変更禁止!');
      mask Help: web(ui_blockhelpmng(gcb));
      mask Display: disp('ATC.DSRVEC')
  SubSystem1627 : Subsystem  TreatAsAtomicUnit=on  mask(***ATC.DSRVEC***){bd_version=1.01.A, r0=DSM19, r1=-1, r2=, r3=, r4=, r5=, r6=, r7=, r8=, r9=, c0=, c1=, c2=, c3=, c4=, c5=, c6=, c7=, c8=, c9=}
      mask prompts: bd_version "Version"
      mask help: これはAutoCからのブロックです．編集禁止．
      settings: OpenFcn=warndlg('変更禁止!');
      mask Help: web(ui_blockhelpmng(gcb));
      mask Display: disp('ATC.DSRVEC')
  Block4357 : Outport
  wires:
  Block4354/1 -> Block4356/1
  Block4356/1 -> Block4357/1
  SubSystem1625/1(Output) -> Block4356/2
  SubSystem1626/1(Output) -> Block4356/3
  SubSystem1627/1(Output) -> Block4356/4
  Block4355/1 -> SubSystem1625/1(element), SubSystem1626/1(element), SubSystem1627/1(element)

== system lib_all/SubSystem1593/SubSystem1595/SubSystem1604/SubSystem1628/SubSystem1629 ==
  note: ***編集禁止***
  element : Inport
  Inside_DSR : DataStoreRead  DataStoreName=DSM5
  Selector : Selector  IndexOptions=Index vector (port)  Indices=[1 3]  OutputSizes=1
  Output : Outport
  wires:
  Selector/1 -> Output/1
  element/1 -> Selector/2
  Inside_DSR/1 -> Selector/1

== system lib_all/SubSystem1593/SubSystem1595/SubSystem1604/SubSystem1628/SubSystem1630 ==
  Block4365 : Inport
  Block4366 : Inport  Port=2
  Block4367 : MultiPortSwitch  zeroidx=off  RndMeth=Zero  SaturateOnIntegerOverflow=on  InputSameDT=on
  SubSystem1631 : Subsystem  TreatAsAtomicUnit=on  mask(***ATC.DSRVEC***){bd_version=1.01.A, r0=DSM7, r1=-1, r2=, r3=, r4=, r5=, r6=, r7=, r8=, r9=, c0=, c1=, c2=, c3=, c4=, c5=, c6=, c7=, c8=, c9=}
      mask prompts: bd_version "Version"
      mask help: これはAutoCからのブロックです．編集禁止．
      settings: OpenFcn=warndlg('変更禁止!');
      mask Help: web(ui_blockhelpmng(gcb));
      mask Display: disp('ATC.DSRVEC')
  SubSystem1632 : Subsystem  TreatAsAtomicUnit=on  mask(***ATC.DSRVEC***){bd_version=1.01.A, r0=DSM6, r1=-1, r2=, r3=, r4=, r5=, r6=, r7=, r8=, r9=, c0=, c1=, c2=, c3=, c4=, c5=, c6=, c7=, c8=, c9=}
      mask prompts: bd_version "Version"
      mask help: これはAutoCからのブロックです．編集禁止．
      settings: OpenFcn=warndlg('変更禁止!');
      mask Help: web(ui_blockhelpmng(gcb));
      mask Display: disp('ATC.DSRVEC')
  SubSystem1633 : Subsystem  TreatAsAtomicUnit=on  mask(***ATC.DSRVEC***){bd_version=1.01.A, r0=DSM19, r1=-1, r2=, r3=, r4=, r5=, r6=, r7=, r8=, r9=, c0=, c1=, c2=, c3=, c4=, c5=, c6=, c7=, c8=, c9=}
      mask prompts: bd_version "Version"
      mask help: これはAutoCからのブロックです．編集禁止．
      settings: OpenFcn=warndlg('変更禁止!');
      mask Help: web(ui_blockhelpmng(gcb));
      mask Display: disp('ATC.DSRVEC')
  Block4368 : Outport
  wires:
  Block4365/1 -> Block4367/1
  Block4367/1 -> Block4368/1
  SubSystem1631/1(Output) -> Block4367/2
  SubSystem1632/1(Output) -> Block4367/3
  SubSystem1633/1(Output) -> Block4367/4
  Block4366/1 -> SubSystem1631/1(element), SubSystem1632/1(element), SubSystem1633/1(element)

== system lib_all/SubSystem1593/SubSystem1595/SubSystem1604/SubSystem1628/SubSystem1634 ==
  Block4369 : Inport
  Block4370 : Inport  Port=2
  Block4371 : MultiPortSwitch  zeroidx=off  RndMeth=Zero  SaturateOnIntegerOverflow=on  InputSameDT=on
  SubSystem1635 : Subsystem  TreatAsAtomicUnit=on  mask(***ATC.DSRVEC***){bd_version=1.01.A, r0=DSM7, r1=-1, r2=, r3=, r4=, r5=, r6=, r7=, r8=, r9=, c0=, c1=, c2=, c3=, c4=, c5=, c6=, c7=, c8=, c9=}
      mask prompts: bd_version "Version"
      mask help: これはAutoCからのブロックです．編集禁止．
      settings: OpenFcn=warndlg('変更禁止!');
      mask Help: web(ui_blockhelpmng(gcb));
      mask Display: disp('ATC.DSRVEC')
  SubSystem1636 : Subsystem  TreatAsAtomicUnit=on  mask(***ATC.DSRVEC***){bd_version=1.01.A, r0=DSM6, r1=-1, r2=, r3=, r4=, r5=, r6=, r7=, r8=, r9=, c0=, c1=, c2=, c3=, c4=, c5=, c6=, c7=, c8=, c9=}
      mask prompts: bd_version "Version"
      mask help: これはAutoCからのブロックです．編集禁止．
      settings: OpenFcn=warndlg('変更禁止!');
      mask Help: web(ui_blockhelpmng(gcb));
      mask Display: disp('ATC.DSRVEC')
  SubSystem1637 : Subsystem  TreatAsAtomicUnit=on  mask(***ATC.DSRVEC***){bd_version=1.01.A, r0=DSM19, r1=-1, r2=, r3=, r4=, r5=, r6=, r7=, r8=, r9=, c0=, c1=, c2=, c3=, c4=, c5=, c6=, c7=, c8=, c9=}
      mask prompts: bd_version "Version"
      mask help: これはAutoCからのブロックです．編集禁止．
      settings: OpenFcn=warndlg('変更禁止!');
      mask Help: web(ui_blockhelpmng(gcb));
      mask Display: disp('ATC.DSRVEC')
  Block4372 : Outport
  wires:
  Block4369/1 -> Block4371/1
  Block4371/1 -> Block4372/1
  SubSystem1635/1(Output) -> Block4371/2
  SubSystem1636/1(Output) -> Block4371/3
  SubSystem1637/1(Output) -> Block4371/4
  Block4370/1 -> SubSystem1635/1(element), SubSystem1636/1(element), SubSystem1637/1(element)

== system lib_all/SubSystem1593/SubSystem1595/SubSystem1604/SubSystem1638/SubSystem1639 ==
  note: ***編集禁止***
  element : Inport
  Inside_DSR : DataStoreRead  DataStoreName=DSM3
  Selector : Selector  IndexOptions=Index vector (port)  Indices=[1 3]  OutputSizes=1
  Output : Outport
  wires:
  Selector/1 -> Output/1
  element/1 -> Selector/2
  Inside_DSR/1 -> Selector/1

== system lib_all/SubSystem1593/SubSystem1595/SubSystem1604/SubSystem1638/SubSystem1640 ==
  Block4380 : Inport
  Block4381 : Inport  Port=2
  Block4382 : MultiPortSwitch  zeroidx=off  RndMeth=Zero  SaturateOnIntegerOverflow=on  InputSameDT=on
  SubSystem1641 : Subsystem  TreatAsAtomicUnit=on  mask(***ATC.DSRVEC***){bd_version=1.01.A, r0=DSM7, r1=-1, r2=, r3=, r4=, r5=, r6=, r7=, r8=, r9=, c0=, c1=, c2=, c3=, c4=, c5=, c6=, c7=, c8=, c9=}
      mask prompts: bd_version "Version"
      mask help: これはAutoCからのブロックです．編集禁止．
      settings: OpenFcn=warndlg('変更禁止!');
      mask Help: web(ui_blockhelpmng(gcb));
      mask Display: disp('ATC.DSRVEC')
  SubSystem1642 : Subsystem  TreatAsAtomicUnit=on  mask(***ATC.DSRVEC***){bd_version=1.01.A, r0=DSM6, r1=-1, r2=, r3=, r4=, r5=, r6=, r7=, r8=, r9=, c0=, c1=, c2=, c3=, c4=, c5=, c6=, c7=, c8=, c9=}
      mask prompts: bd_version "Version"
      mask help: これはAutoCからのブロックです．編集禁止．
      settings: OpenFcn=warndlg('変更禁止!');
      mask Help: web(ui_blockhelpmng(gcb));
      mask Display: disp('ATC.DSRVEC')
  SubSystem1643 : Subsystem  TreatAsAtomicUnit=on  mask(***ATC.DSRVEC***){bd_version=1.01.A, r0=DSM19, r1=-1, r2=, r3=, r4=, r5=, r6=, r7=, r8=, r9=, c0=, c1=, c2=, c3=, c4=, c5=, c6=, c7=, c8=, c9=}
      mask prompts: bd_version "Version"
      mask help: これはAutoCからのブロックです．編集禁止．
      settings: OpenFcn=warndlg('変更禁止!');
      mask Help: web(ui_blockhelpmng(gcb));
      mask Display: disp('ATC.DSRVEC')
  Block4383 : Outport
  wires:
  Block4380/1 -> Block4382/1
  Block4382/1 -> Block4383/1
  SubSystem1641/1(Output) -> Block4382/2
  SubSystem1642/1(Output) -> Block4382/3
  SubSystem1643/1(Output) -> Block4382/4
  Block4381/1 -> SubSystem1641/1(element), SubSystem1642/1(element), SubSystem1643/1(element)

== system lib_all/SubSystem1593/SubSystem1595/SubSystem1604/SubSystem1638/SubSystem1644 ==
  Block4384 : Inport
  Block4385 : Inport  Port=2
  Block4386 : MultiPortSwitch  zeroidx=off  RndMeth=Zero  SaturateOnIntegerOverflow=on  InputSameDT=on
  SubSystem1645 : Subsystem  TreatAsAtomicUnit=on  mask(***ATC.DSRVEC***){bd_version=1.01.A, r0=DSM7, r1=-1, r2=, r3=, r4=, r5=, r6=, r7=, r8=, r9=, c0=, c1=, c2=, c3=, c4=, c5=, c6=, c7=, c8=, c9=}
      mask prompts: bd_version "Version"
      mask help: これはAutoCからのブロックです．編集禁止．
      settings: OpenFcn=warndlg('変更禁止!');
      mask Help: web(ui_blockhelpmng(gcb));
      mask Display: disp('ATC.DSRVEC')
  SubSystem1646 : Subsystem  TreatAsAtomicUnit=on  mask(***ATC.DSRVEC***){bd_version=1.01.A, r0=DSM6, r1=-1, r2=, r3=, r4=, r5=, r6=, r7=, r8=, r9=, c0=, c1=, c2=, c3=, c4=, c5=, c6=, c7=, c8=, c9=}
      mask prompts: bd_version "Version"
      mask help: これはAutoCからのブロックです．編集禁止．
      settings: OpenFcn=warndlg('変更禁止!');
      mask Help: web(ui_blockhelpmng(gcb));
      mask Display: disp('ATC.DSRVEC')
  SubSystem1647 : Subsystem  TreatAsAtomicUnit=on  mask(***ATC.DSRVEC***){bd_version=1.01.A, r0=DSM19, r1=-1, r2=, r3=, r4=, r5=, r6=, r7=, r8=, r9=, c0=, c1=, c2=, c3=, c4=, c5=, c6=, c7=, c8=, c9=}
      mask prompts: bd_version "Version"
      mask help: これはAutoCからのブロックです．編集禁止．
      settings: OpenFcn=warndlg('変更禁止!');
      mask Help: web(ui_blockhelpmng(gcb));
      mask Display: disp('ATC.DSRVEC')
  Block4387 : Outport
  wires:
  Block4384/1 -> Block4386/1
  Block4386/1 -> Block4387/1
  SubSystem1645/1(Output) -> Block4386/2
  SubSystem1646/1(Output) -> Block4386/3
  SubSystem1647/1(Output) -> Block4386/4
  Block4385/1 -> SubSystem1645/1(element), SubSystem1646/1(element), SubSystem1647/1(element)

== system lib_all/SubSystem1593/SubSystem1648/SubSystem1650/SubSystem1651/SubSystem1652 ==
  note: ***編集禁止***
  In1 : Inport
  In2 : Inport  Port=2
  RO1 : RelationalOperator  Operator===  InputSameDT=on
  single1 : DataTypeConversion  OutDataTypeStr=single  SaturateOnIntegerOverflow=on  RndMeth=Zero
  single2 : DataTypeConversion  OutDataTypeStr=single  SaturateOnIntegerOverflow=on  RndMeth=Zero
  Out1 : Outport
  wires:
  RO1/1 -> Out1/1
  In2/1 -> single2/1
  In1/1 -> single1/1
  single1/1 -> RO1/1
  single2/1 -> RO1/2
